simulink model "VirtualADAPT"  (Simulink 8.9)
settings: Solver=ode45  StartTime=0.0  StopTime=inf  RelTol=1e-3  EnableMultiTasking=on
PostLoadFcn:
  | FaultInjectionGUIHandle = FaultInjectionGUI;
InitFcn:
  | VirtualADAPTDataStructure
undefined symbols (not defined in the file or in its folder): ln
libraries: simulink x363
(part 2 of 26)

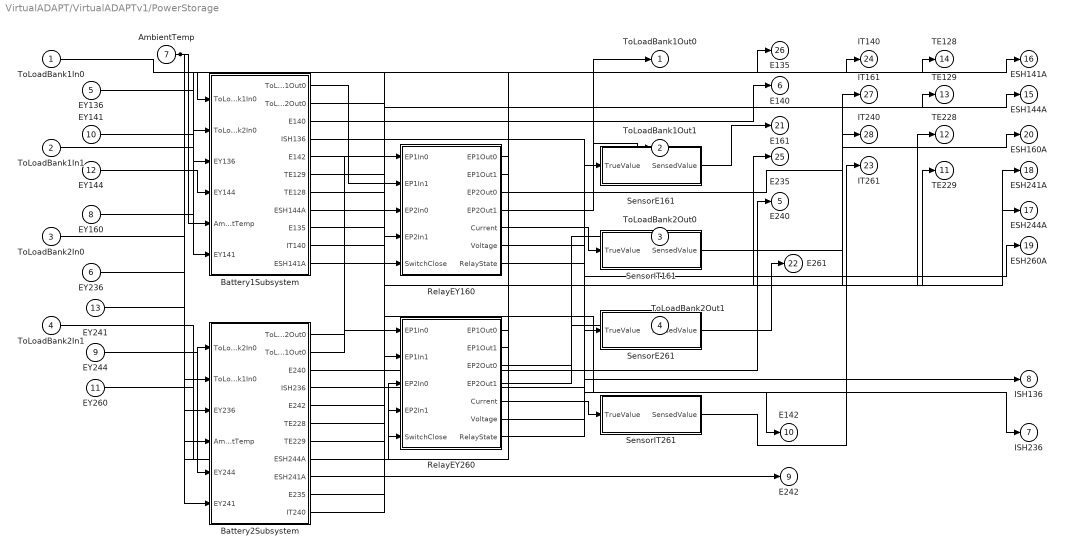
== system VirtualADAPT/VirtualADAPTv1/PowerStorage ==
  ToLoadBank1In0 : Inport
  ToLoadBank1In1 : Inport  Port=2
  ToLoadBank2In0 : Inport  Port=3
  ToLoadBank2In1 : Inport  Port=4
  EY136 : Inport  Port=5
  EY236 : Inport  Port=6
  AmbientTemp : Inport  Port=7
  EY160 : Inport  Port=8
  EY244 : Inport  Port=9
  EY141 : Inport  Port=10
  EY260 : Inport  Port=11
  EY144 : Inport  Port=12
  EY241 : Inport  Port=13
  Battery1Subsystem : Subsystem
  Battery2Subsystem : Subsystem
  RelayEY160 : Subsystem
  RelayEY260 : Subsystem
  SensorE161 : Subsystem
  SensorE261 : Subsystem
  SensorIT161 : Subsystem
  SensorIT261 : Subsystem
  ToLoadBank1Out0 : Outport
  ToLoadBank1Out1 : Outport  Port=2
  ToLoadBank2Out0 : Outport  Port=3
  ToLoadBank2Out1 : Outport  Port=4
  E240 : Outport  Port=5
  E140 : Outport  Port=6
  ISH236 : Outport  Port=7
  ISH136 : Outport  Port=8
  E242 : Outport  Port=9
  E142 : Outport  Port=10
  TE229 : Outport  Port=11
  TE228 : Outport  Port=12
  TE129 : Outport  Port=13
  TE128 : Outport  Port=14
  ESH144A : Outport  Port=15
  ESH141A : Outport  Port=16
  ESH244A : Outport  Port=17
  ESH241A : Outport  Port=18
  ESH260A : Outport  Port=19
  ESH160A : Outport  Port=20
  E161 : Outport  Port=21
  E261 : Outport  Port=22
  IT261 : Outport  Port=23
  IT140 : Outport  Port=24
  E235 : Outport  Port=25
  E135 : Outport  Port=26
  IT161 : Outport  Port=27
  IT240 : Outport  Port=28
  wires:
  Battery1Subsystem/1(ToLoadBank1Out0) -> RelayEY160/2(EP1In1)
  RelayEY160/2(EP1Out1) -> Battery1Subsystem/1(ToLoadBank1In0)
  Battery2Subsystem/1(ToLoadBank2Out0) -> RelayEY260/1(EP1In0)
  RelayEY260/1(EP1Out0) -> Battery2Subsystem/1(ToLoadBank2In0)
  Battery1Subsystem/2(ToLoadBank2Out0) -> RelayEY260/2(EP1In1)
  RelayEY260/2(EP1Out1) -> Battery1Subsystem/2(ToLoadBank2In0)
  RelayEY160/3(EP2Out0) -> ToLoadBank1Out0/1
  ToLoadBank1In0/1 -> RelayEY160/3(EP2In0)
  RelayEY160/4(EP2Out1) -> ToLoadBank1Out1/1
  ToLoadBank1In1/1 -> RelayEY160/4(EP2In1)
  Battery2Subsystem/2(ToLoadBank1Out0) -> RelayEY160/1(EP1In0)
  RelayEY160/1(EP1Out0) -> Battery2Subsystem/2(ToLoadBank1In0)
  RelayEY260/3(EP2Out0) -> ToLoadBank2Out0/1
  ToLoadBank2In0/1 -> RelayEY260/3(EP2In0)
  RelayEY260/4(EP2Out1) -> ToLoadBank2Out1/1
  ToLoadBank2In1/1 -> RelayEY260/4(EP2In1)
  Battery1Subsystem/5(E142) -> E142/1
  Battery2Subsystem/3(E240) -> E240/1
  SensorE161/1(SensedValue) -> E161/1
  Battery1Subsystem/8(ESH144A) -> ESH144A/1
  Battery1Subsystem/11(ESH141A) -> ESH141A/1
  EY260/1 -> RelayEY260/5(SwitchClose)
  SensorIT261/1(SensedValue) -> IT261/1
  EY236/1 -> Battery2Subsystem/3(EY236)
  EY136/1 -> Battery1Subsystem/3(EY136)
  Battery2Subsystem/5(E242) -> E242/1
  EY144/1 -> Battery1Subsystem/4(EY144)
  RelayEY160/5(Current) -> SensorIT161/1(TrueValue)
  RelayEY160/6(Voltage) -> SensorE161/1(TrueValue)
  RelayEY160/7(RelayState) -> ESH160A/1
  RelayEY260/5(Current) -> SensorIT261/1(TrueValue)
  RelayEY260/6(Voltage) -> SensorE261/1(TrueValue)
  RelayEY260/7(RelayState) -> ESH260A/1
  Battery2Subsystem/10(E235) -> E235/1
  SensorIT161/1(SensedValue) -> IT161/1
  SensorE261/1(SensedValue) -> E261/1
  Battery1Subsystem/10(IT140) -> IT140/1
  EY160/1 -> RelayEY160/5(SwitchClose)
  EY141/1 -> Battery1Subsystem/6(EY141)
  Battery1Subsystem/3(E140) -> E140/1
  Battery2Subsystem/11(IT240) -> IT240/1
  EY241/1 -> Battery2Subsystem/6(EY241)
  Battery1Subsystem/4(ISH136) -> ISH136/1
  Battery2Subsystem/4(ISH236) -> ISH236/1
  EY244/1 -> Battery2Subsystem/5(EY244)
  AmbientTemp/1 -> Battery2Subsystem/4(AmbientTemp), Battery1Subsystem/5(AmbientTemp)
  Battery1Subsystem/9(E135) -> E135/1
  Battery2Subsystem/8(ESH244A) -> ESH244A/1
  Battery2Subsystem/9(ESH241A) -> ESH241A/1
  Battery2Subsystem/7(TE229) -> TE229/1
  Battery2Subsystem/6(TE228) -> TE228/1
  Battery1Subsystem/6(TE129) -> TE129/1
  Battery1Subsystem/7(TE128) -> TE128/1

== system VirtualADAPT/VirtualADAPTv1/SensorTE133 ==
  TrueValue : Inport
  MSe : Subsystem  mask{FP=Nominal, FT=0, FM=0}
      mask prompts: FP "Fault Profile:"; FT "Fault Injection Time:"; FM "Fault Magnitude/Slope/Bias/StuckAtValue"
      mask TypeOptions FP: Nominal | Abrupt | Incipient | Bias | StuckAt
      mask Display: image(imread('MSe.png','BackgroundColor',[1 1 1]))
  R : Subsystem  mask{R=1, FP=Nominal, FT=0, FM=0}
      mask prompts: R "Value of R:"; FP "Fault Profile:"; FT "Fault Injection Time:"; FM "Fault Magnitude/Slope"
      mask TypeOptions FP: Nominal | Abrupt | Incipient
      mask Display: image(imread('R.png','BackgroundColor',[1 1 1]))
  TempBias : Subsystem  mask{Constant=0, FP=Nominal, FT=0, FM=0}
      mask prompts: Constant "Value of Constant:"; FP "Fault Profile:"; FT "Fault Injection Time:"; FM "Fault Magnitude/Slope"
      mask TypeOptions FP: Nominal | Abrupt | Incipient
      mask Display: image(imread('Constant.png','BackgroundColor',[1 1 1]))
  TrueValueMod : Subsystem
      mask Display: image(imread('fx.png','BackgroundColor',[1 1 1]))
  Value : Subsystem
      mask Display: image(imread('0.png','BackgroundColor',[1 1 1]))
  SensedValue : Outport
  wires:
  Value/1(y(1)) -> R/1(In)
  R/1(Out) -> Value/1(u(1))
  MSe/1(E) -> Value/2(u(2))
  Value/2(y(2)) -> MSe/1(F)
  TrueValueMod/1(y(1)) -> MSe/2(Se)
  TrueValue/1 -> TrueValueMod/1(u(1))
  TempBias/1(C) -> TrueValueMod/2(u(2))
  Value/3(O) -> SensedValue/1

== system VirtualADAPT/VirtualADAPTv1/AmbientTemp/AmbientTemp ==
  Constant : Constant  Value=Constant
  Fault Inject : Subsystem
  Fault Profile : Constant  Value=FP
  Ramp : simulink/Sources/Ramp  slope=FM  start=FT  X0=Constant
  Step : Step  Time=FT  Before=Constant  After=(1 + FM)*Constant  SampleTime=0
  C : Outport
  wires:
  Fault Inject/1(Out1) -> C/1
  Constant/1 -> Fault Inject/1(Nominal)
  Fault Profile/1 -> Fault Inject/2(Fault Profile)
  Step/1 -> Fault Inject/3(Step)
  Ramp/1 -> Fault Inject/4(Ramp)

== system VirtualADAPT/VirtualADAPTv1/EY136_OPFaultInject/Fault Inject ==
  Nominal : Inport
  Fault Profile : Inport  Port=2
  Step : Inport  Port=3
  Ramp : Inport  Port=4
  Bias : Inport  Port=5
  stuckAt : Inport  Port=6
  Switch1 : MultiPortSwitch  Inputs=5  SaturateOnIntegerOverflow=on  InputSameDT=on
  Out1 : Outport
  wires:
  Nominal/1 -> Switch1/2
  Fault Profile/1 -> Switch1/1
  Step/1 -> Switch1/3
  Ramp/1 -> Switch1/4
  Bias/1 -> Switch1/5
  stuckAt/1 -> Switch1/6
  Switch1/1 -> Out1/1

== system VirtualADAPT/VirtualADAPTv1/EY141_CLFaultInject/Fault Inject ==
  Nominal : Inport
  Fault Profile : Inport  Port=2
  Step : Inport  Port=3
  Ramp : Inport  Port=4
  Bias : Inport  Port=5
  stuckAt : Inport  Port=6
  Switch1 : MultiPortSwitch  Inputs=5  SaturateOnIntegerOverflow=on  InputSameDT=on
  Out1 : Outport
  wires:
  Nominal/1 -> Switch1/2
  Fault Profile/1 -> Switch1/1
  Step/1 -> Switch1/3
  Ramp/1 -> Switch1/4
  Bias/1 -> Switch1/5
  stuckAt/1 -> Switch1/6
  Switch1/1 -> Out1/1

== system VirtualADAPT/VirtualADAPTv1/EY144_CLFaultInject/Fault Inject ==
  Nominal : Inport
  Fault Profile : Inport  Port=2
  Step : Inport  Port=3
  Ramp : Inport  Port=4
  Bias : Inport  Port=5
  stuckAt : Inport  Port=6
  Switch1 : MultiPortSwitch  Inputs=5  SaturateOnIntegerOverflow=on  InputSameDT=on
  Out1 : Outport
  wires:
  Nominal/1 -> Switch1/2
  Fault Profile/1 -> Switch1/1
  Step/1 -> Switch1/3
  Ramp/1 -> Switch1/4
  Bias/1 -> Switch1/5
  stuckAt/1 -> Switch1/6
  Switch1/1 -> Out1/1

== system VirtualADAPT/VirtualADAPTv1/EY160_CLFaultInject/Fault Inject ==
  Nominal : Inport
  Fault Profile : Inport  Port=2
  Step : Inport  Port=3
  Ramp : Inport  Port=4
  Bias : Inport  Port=5
  stuckAt : Inport  Port=6
  Switch1 : MultiPortSwitch  Inputs=5  SaturateOnIntegerOverflow=on  InputSameDT=on
  Out1 : Outport
  wires:
  Nominal/1 -> Switch1/2
  Fault Profile/1 -> Switch1/1
  Step/1 -> Switch1/3
  Ramp/1 -> Switch1/4
  Bias/1 -> Switch1/5
  stuckAt/1 -> Switch1/6
  Switch1/1 -> Out1/1

== system VirtualADAPT/VirtualADAPTv1/EY170_CLFaultInject/Fault Inject ==
  Nominal : Inport
  Fault Profile : Inport  Port=2
  Step : Inport  Port=3
  Ramp : Inport  Port=4
  Bias : Inport  Port=5
  stuckAt : Inport  Port=6
  Switch1 : MultiPortSwitch  Inputs=5  SaturateOnIntegerOverflow=on  InputSameDT=on
  Out1 : Outport
  wires:
  Nominal/1 -> Switch1/2
  Fault Profile/1 -> Switch1/1
  Step/1 -> Switch1/3
  Ramp/1 -> Switch1/4
  Bias/1 -> Switch1/5
  stuckAt/1 -> Switch1/6
  Switch1/1 -> Out1/1

== system VirtualADAPT/VirtualADAPTv1/EY171_CLFaultInject/Fault Inject ==
  Nominal : Inport
  Fault Profile : Inport  Port=2
  Step : Inport  Port=3
  Ramp : Inport  Port=4
  Bias : Inport  Port=5
  stuckAt : Inport  Port=6
  Switch1 : MultiPortSwitch  Inputs=5  SaturateOnIntegerOverflow=on  InputSameDT=on
  Out1 : Outport
  wires:
  Nominal/1 -> Switch1/2
  Fault Profile/1 -> Switch1/1
  Step/1 -> Switch1/3
  Ramp/1 -> Switch1/4
  Bias/1 -> Switch1/5
  stuckAt/1 -> Switch1/6
  Switch1/1 -> Out1/1

== system VirtualADAPT/VirtualADAPTv1/EY172_CLFaultInject/Fault Inject ==
  Nominal : Inport
  Fault Profile : Inport  Port=2
  Step : Inport  Port=3
  Ramp : Inport  Port=4
  Bias : Inport  Port=5
  stuckAt : Inport  Port=6
  Switch1 : MultiPortSwitch  Inputs=5  SaturateOnIntegerOverflow=on  InputSameDT=on
  Out1 : Outport
  wires:
  Nominal/1 -> Switch1/2
  Fault Profile/1 -> Switch1/1
  Step/1 -> Switch1/3
  Ramp/1 -> Switch1/4
  Bias/1 -> Switch1/5
  stuckAt/1 -> Switch1/6
  Switch1/1 -> Out1/1

== system VirtualADAPT/VirtualADAPTv1/EY173_CLFaultInject/Fault Inject ==
  Nominal : Inport
  Fault Profile : Inport  Port=2
  Step : Inport  Port=3
  Ramp : Inport  Port=4
  Bias : Inport  Port=5
  stuckAt : Inport  Port=6
  Switch1 : MultiPortSwitch  Inputs=5  SaturateOnIntegerOverflow=on  InputSameDT=on
  Out1 : Outport
  wires:
  Nominal/1 -> Switch1/2
  Fault Profile/1 -> Switch1/1
  Step/1 -> Switch1/3
  Ramp/1 -> Switch1/4
  Bias/1 -> Switch1/5
  stuckAt/1 -> Switch1/6
  Switch1/1 -> Out1/1

== system VirtualADAPT/VirtualADAPTv1/EY174_CLFaultInject/Fault Inject ==
  Nominal : Inport
  Fault Profile : Inport  Port=2
  Step : Inport  Port=3
  Ramp : Inport  Port=4
  Bias : Inport  Port=5
  stuckAt : Inport  Port=6
  Switch1 : MultiPortSwitch  Inputs=5  SaturateOnIntegerOverflow=on  InputSameDT=on
  Out1 : Outport
  wires:
  Nominal/1 -> Switch1/2
  Fault Profile/1 -> Switch1/1
  Step/1 -> Switch1/3
  Ramp/1 -> Switch1/4
  Bias/1 -> Switch1/5
  stuckAt/1 -> Switch1/6
  Switch1/1 -> Out1/1

== system VirtualADAPT/VirtualADAPTv1/EY175_CLFaultInject/Fault Inject ==
  Nominal : Inport
  Fault Profile : Inport  Port=2
  Step : Inport  Port=3
  Ramp : Inport  Port=4
  Bias : Inport  Port=5
  stuckAt : Inport  Port=6
  Switch1 : MultiPortSwitch  Inputs=5  SaturateOnIntegerOverflow=on  InputSameDT=on
  Out1 : Outport
  wires:
  Nominal/1 -> Switch1/2
  Fault Profile/1 -> Switch1/1
  Step/1 -> Switch1/3
  Ramp/1 -> Switch1/4
  Bias/1 -> Switch1/5
  stuckAt/1 -> Switch1/6
  Switch1/1 -> Out1/1

== system VirtualADAPT/VirtualADAPTv1/EY183_CLFaultInject/Fault Inject ==
  Nominal : Inport
  Fault Profile : Inport  Port=2
  Step : Inport  Port=3
  Ramp : Inport  Port=4
  Bias : Inport  Port=5
  stuckAt : Inport  Port=6
  Switch1 : MultiPortSwitch  Inputs=5  SaturateOnIntegerOverflow=on  InputSameDT=on
  Out1 : Outport
  wires:
  Nominal/1 -> Switch1/2
  Fault Profile/1 -> Switch1/1
  Step/1 -> Switch1/3
  Ramp/1 -> Switch1/4
  Bias/1 -> Switch1/5
  stuckAt/1 -> Switch1/6
  Switch1/1 -> Out1/1

== system VirtualADAPT/VirtualADAPTv1/EY184_CLFaultInject/Fault Inject ==
  Nominal : Inport
  Fault Profile : Inport  Port=2
  Step : Inport  Port=3
  Ramp : Inport  Port=4
  Bias : Inport  Port=5
  stuckAt : Inport  Port=6
  Switch1 : MultiPortSwitch  Inputs=5  SaturateOnIntegerOverflow=on  InputSameDT=on
  Out1 : Outport
  wires:
  Nominal/1 -> Switch1/2
  Fault Profile/1 -> Switch1/1
  Step/1 -> Switch1/3
  Ramp/1 -> Switch1/4
  Bias/1 -> Switch1/5
  stuckAt/1 -> Switch1/6
  Switch1/1 -> Out1/1

== system VirtualADAPT/VirtualADAPTv1/EY236_OPFaultInject/Fault Inject ==
  Nominal : Inport
  Fault Profile : Inport  Port=2
  Step : Inport  Port=3
  Ramp : Inport  Port=4
  Bias : Inport  Port=5
  stuckAt : Inport  Port=6
  Switch1 : MultiPortSwitch  Inputs=5  SaturateOnIntegerOverflow=on  InputSameDT=on
  Out1 : Outport
  wires:
  Nominal/1 -> Switch1/2
  Fault Profile/1 -> Switch1/1
  Step/1 -> Switch1/3
  Ramp/1 -> Switch1/4
  Bias/1 -> Switch1/5
  stuckAt/1 -> Switch1/6
  Switch1/1 -> Out1/1

== system VirtualADAPT/VirtualADAPTv1/EY241_CLFaultInject/Fault Inject ==
  Nominal : Inport
  Fault Profile : Inport  Port=2
  Step : Inport  Port=3
  Ramp : Inport  Port=4
  Bias : Inport  Port=5
  stuckAt : Inport  Port=6
  Switch1 : MultiPortSwitch  Inputs=5  SaturateOnIntegerOverflow=on  InputSameDT=on
  Out1 : Outport
  wires:
  Nominal/1 -> Switch1/2
  Fault Profile/1 -> Switch1/1
  Step/1 -> Switch1/3
  Ramp/1 -> Switch1/4
  Bias/1 -> Switch1/5
  stuckAt/1 -> Switch1/6
  Switch1/1 -> Out1/1

== system VirtualADAPT/VirtualADAPTv1/EY244_CLFaultInject/Fault Inject ==
  Nominal : Inport
  Fault Profile : Inport  Port=2
  Step : Inport  Port=3
  Ramp : Inport  Port=4
  Bias : Inport  Port=5
  stuckAt : Inport  Port=6
  Switch1 : MultiPortSwitch  Inputs=5  SaturateOnIntegerOverflow=on  InputSameDT=on
  Out1 : Outport
  wires:
  Nominal/1 -> Switch1/2
  Fault Profile/1 -> Switch1/1
  Step/1 -> Switch1/3
  Ramp/1 -> Switch1/4
  Bias/1 -> Switch1/5
  stuckAt/1 -> Switch1/6
  Switch1/1 -> Out1/1

== system VirtualADAPT/VirtualADAPTv1/EY260_CLFaultInject/Fault Inject ==
  Nominal : Inport
  Fault Profile : Inport  Port=2
  Step : Inport  Port=3
  Ramp : Inport  Port=4
  Bias : Inport  Port=5
  stuckAt : Inport  Port=6
  Switch1 : MultiPortSwitch  Inputs=5  SaturateOnIntegerOverflow=on  InputSameDT=on
  Out1 : Outport
  wires:
  Nominal/1 -> Switch1/2
  Fault Profile/1 -> Switch1/1
  Step/1 -> Switch1/3
  Ramp/1 -> Switch1/4
  Bias/1 -> Switch1/5
  stuckAt/1 -> Switch1/6
  Switch1/1 -> Out1/1

== system VirtualADAPT/VirtualADAPTv1/EY270_CLFaultInject/Fault Inject ==
  Nominal : Inport
  Fault Profile : Inport  Port=2
  Step : Inport  Port=3
  Ramp : Inport  Port=4
  Bias : Inport  Port=5
  stuckAt : Inport  Port=6
  Switch1 : MultiPortSwitch  Inputs=5  SaturateOnIntegerOverflow=on  InputSameDT=on
  Out1 : Outport
  wires:
  Nominal/1 -> Switch1/2
  Fault Profile/1 -> Switch1/1
  Step/1 -> Switch1/3
  Ramp/1 -> Switch1/4
  Bias/1 -> Switch1/5
  stuckAt/1 -> Switch1/6
  Switch1/1 -> Out1/1

== system VirtualADAPT/VirtualADAPTv1/EY271_CLFaultInject/Fault Inject ==
  Nominal : Inport
  Fault Profile : Inport  Port=2
  Step : Inport  Port=3
  Ramp : Inport  Port=4
  Bias : Inport  Port=5
  stuckAt : Inport  Port=6
  Switch1 : MultiPortSwitch  Inputs=5  SaturateOnIntegerOverflow=on  InputSameDT=on
  Out1 : Outport
  wires:
  Nominal/1 -> Switch1/2
  Fault Profile/1 -> Switch1/1
  Step/1 -> Switch1/3
  Ramp/1 -> Switch1/4
  Bias/1 -> Switch1/5
  stuckAt/1 -> Switch1/6
  Switch1/1 -> Out1/1

== system VirtualADAPT/VirtualADAPTv1/EY272_CLFaultInject/Fault Inject ==
  Nominal : Inport
  Fault Profile : Inport  Port=2
  Step : Inport  Port=3
  Ramp : Inport  Port=4
  Bias : Inport  Port=5
  stuckAt : Inport  Port=6
  Switch1 : MultiPortSwitch  Inputs=5  SaturateOnIntegerOverflow=on  InputSameDT=on
  Out1 : Outport
  wires:
  Nominal/1 -> Switch1/2
  Fault Profile/1 -> Switch1/1
  Step/1 -> Switch1/3
  Ramp/1 -> Switch1/4
  Bias/1 -> Switch1/5
  stuckAt/1 -> Switch1/6
  Switch1/1 -> Out1/1

== system VirtualADAPT/VirtualADAPTv1/EY273_CLFaultInject/Fault Inject ==
  Nominal : Inport
  Fault Profile : Inport  Port=2
  Step : Inport  Port=3
  Ramp : Inport  Port=4
  Bias : Inport  Port=5
  stuckAt : Inport  Port=6
  Switch1 : MultiPortSwitch  Inputs=5  SaturateOnIntegerOverflow=on  InputSameDT=on
  Out1 : Outport
  wires:
  Nominal/1 -> Switch1/2
  Fault Profile/1 -> Switch1/1
  Step/1 -> Switch1/3
  Ramp/1 -> Switch1/4
  Bias/1 -> Switch1/5
  stuckAt/1 -> Switch1/6
  Switch1/1 -> Out1/1

== system VirtualADAPT/VirtualADAPTv1/EY274_CLFaultInject/Fault Inject ==
  Nominal : Inport
  Fault Profile : Inport  Port=2
  Step : Inport  Port=3
  Ramp : Inport  Port=4
  Bias : Inport  Port=5
  stuckAt : Inport  Port=6
  Switch1 : MultiPortSwitch  Inputs=5  SaturateOnIntegerOverflow=on  InputSameDT=on
  Out1 : Outport
  wires:
  Nominal/1 -> Switch1/2
  Fault Profile/1 -> Switch1/1
  Step/1 -> Switch1/3
  Ramp/1 -> Switch1/4
  Bias/1 -> Switch1/5
  stuckAt/1 -> Switch1/6
  Switch1/1 -> Out1/1

== system VirtualADAPT/VirtualADAPTv1/EY275_CLFaultInject/Fault Inject ==
  Nominal : Inport
  Fault Profile : Inport  Port=2
  Step : Inport  Port=3
  Ramp : Inport  Port=4
  Bias : Inport  Port=5
  stuckAt : Inport  Port=6
  Switch1 : MultiPortSwitch  Inputs=5  SaturateOnIntegerOverflow=on  InputSameDT=on
  Out1 : Outport
  wires:
  Nominal/1 -> Switch1/2
  Fault Profile/1 -> Switch1/1
  Step/1 -> Switch1/3
  Ramp/1 -> Switch1/4
  Bias/1 -> Switch1/5
  stuckAt/1 -> Switch1/6
  Switch1/1 -> Out1/1

== system VirtualADAPT/VirtualADAPTv1/EY283_CLFaultInject/Fault Inject ==
  Nominal : Inport
  Fault Profile : Inport  Port=2
  Step : Inport  Port=3
  Ramp : Inport  Port=4
  Bias : Inport  Port=5
  stuckAt : Inport  Port=6
  Switch1 : MultiPortSwitch  Inputs=5  SaturateOnIntegerOverflow=on  InputSameDT=on
  Out1 : Outport
  wires:
  Nominal/1 -> Switch1/2
  Fault Profile/1 -> Switch1/1
  Step/1 -> Switch1/3
  Ramp/1 -> Switch1/4
  Bias/1 -> Switch1/5
  stuckAt/1 -> Switch1/6
  Switch1/1 -> Out1/1

== system VirtualADAPT/VirtualADAPTv1/EY284_CLFaultInject/Fault Inject ==
  Nominal : Inport
  Fault Profile : Inport  Port=2
  Step : Inport  Port=3
  Ramp : Inport  Port=4
  Bias : Inport  Port=5
  stuckAt : Inport  Port=6
  Switch1 : MultiPortSwitch  Inputs=5  SaturateOnIntegerOverflow=on  InputSameDT=on
  Out1 : Outport
  wires:
  Nominal/1 -> Switch1/2
  Fault Profile/1 -> Switch1/1
  Step/1 -> Switch1/3
  Ramp/1 -> Switch1/4
  Bias/1 -> Switch1/5
  stuckAt/1 -> Switch1/6
  Switch1/1 -> Out1/1

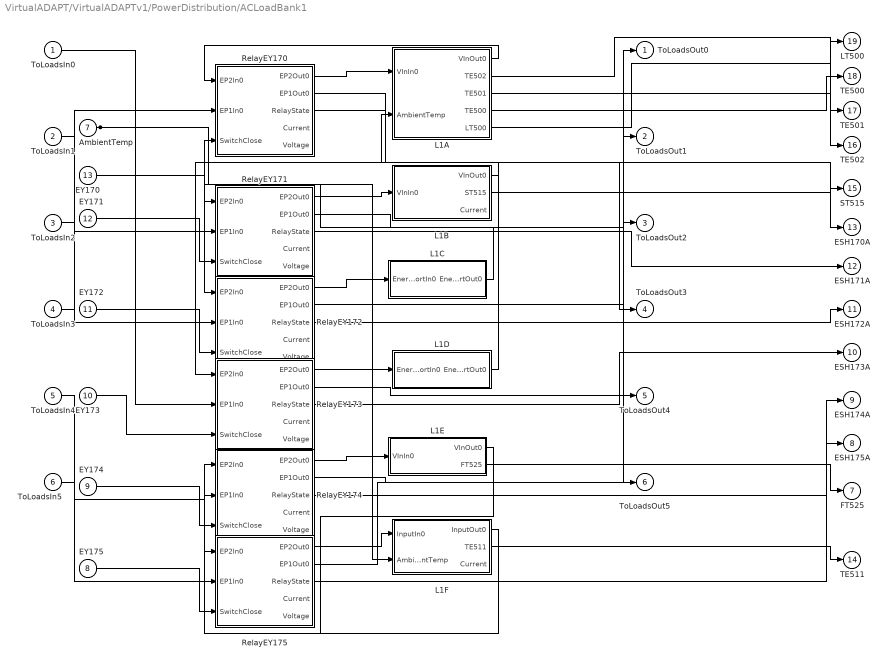
== system VirtualADAPT/VirtualADAPTv1/PowerDistribution/ACLoadBank1 ==
  ToLoadsIn0 : Inport
  ToLoadsIn1 : Inport  Port=2
  ToLoadsIn2 : Inport  Port=3
  ToLoadsIn3 : Inport  Port=4
  ToLoadsIn4 : Inport  Port=5
  ToLoadsIn5 : Inport  Port=6
  AmbientTemp : Inport  Port=7
  EY175 : Inport  Port=8
  EY174 : Inport  Port=9
  EY173 : Inport  Port=10
  EY172 : Inport  Port=11
  EY171 : Inport  Port=12
  EY170 : Inport  Port=13
  L1A : Subsystem
  L1B : Subsystem
  L1C : Subsystem
  L1D : Subsystem
  L1E : Subsystem
  L1F : Subsystem
  RelayEY170 : Subsystem
  RelayEY171 : Subsystem
  RelayEY172 : Subsystem
  RelayEY173 : Subsystem
  RelayEY174 : Subsystem
  RelayEY175 : Subsystem
  ToLoadsOut0 : Outport
  ToLoadsOut1 : Outport  Port=2
  ToLoadsOut2 : Outport  Port=3
  ToLoadsOut3 : Outport  Port=4
  ToLoadsOut4 : Outport  Port=5
  ToLoadsOut5 : Outport  Port=6
  FT525 : Outport  Port=7
  ESH175A : Outport  Port=8
  ESH174A : Outport  Port=9
  ESH173A : Outport  Port=10
  ESH172A : Outport  Port=11
  ESH171A : Outport  Port=12
  ESH170A : Outport  Port=13
  TE511 : Outport  Port=14
  ST515 : Outport  Port=15
  TE502 : Outport  Port=16
  TE501 : Outport  Port=17
  TE500 : Outport  Port=18
  LT500 : Outport  Port=19
  wires:
  RelayEY172/1(EP2Out0) -> L1C/1(EnergyPortIn0)
  L1C/1(EnergyPortOut0) -> RelayEY172/1(EP2In0)
  RelayEY173/1(EP2Out0) -> L1D/1(EnergyPortIn0)
  L1D/1(EnergyPortOut0) -> RelayEY173/1(EP2In0)
  RelayEY174/1(EP2Out0) -> L1E/1(VInIn0)
  L1E/1(VInOut0) -> RelayEY174/1(EP2In0)
  RelayEY171/1(EP2Out0) -> L1B/1(VInIn0)
  L1B/1(VInOut0) -> RelayEY171/1(EP2In0)
  ToLoadsIn0/1 -> RelayEY173/2(EP1In0)
  RelayEY173/2(EP1Out0) -> ToLoadsOut0/1
  RelayEY175/1(EP2Out0) -> L1F/1(InputIn0)
  L1F/1(InputOut0) -> RelayEY175/1(EP2In0)
  RelayEY170/1(EP2Out0) -> L1A/1(VInIn0)
  L1A/1(VInOut0) -> RelayEY170/1(EP2In0)
  ToLoadsIn1/1 -> RelayEY172/2(EP1In0)
  RelayEY172/2(EP1Out0) -> ToLoadsOut1/1
  ToLoadsIn2/1 -> RelayEY171/2(EP1In0)
  RelayEY171/2(EP1Out0) -> ToLoadsOut2/1
  ToLoadsIn3/1 -> RelayEY170/2(EP1In0)
  RelayEY170/2(EP1Out0) -> ToLoadsOut3/1
  ToLoadsIn4/1 -> RelayEY175/2(EP1In0)
  RelayEY175/2(EP1Out0) -> ToLoadsOut4/1
  ToLoadsIn5/1 -> RelayEY174/2(EP1In0)
  RelayEY174/2(EP1Out0) -> ToLoadsOut5/1
  L1E/2(FT525) -> FT525/1
  RelayEY175/3(RelayState) -> ESH175A/1
  RelayEY174/3(RelayState) -> ESH174A/1
  RelayEY173/3(RelayState) -> ESH173A/1
  RelayEY172/3(RelayState) -> ESH172A/1
  RelayEY171/3(RelayState) -> ESH171A/1
  RelayEY170/3(RelayState) -> ESH170A/1
  L1B/2(ST515) -> ST515/1
  AmbientTemp/1 -> L1F/2(AmbientTemp), L1A/2(AmbientTemp)
  L1F/2(TE511) -> TE511/1
  L1A/2(TE502) -> TE502/1
  L1A/3(TE501) -> TE501/1
  L1A/4(TE500) -> TE500/1
  L1A/5(LT500) -> LT500/1
  EY173/1 -> RelayEY173/3(SwitchClose)
  EY172/1 -> RelayEY172/3(SwitchClose)
  EY171/1 -> RelayEY171/3(SwitchClose)
  EY170/1 -> RelayEY170/3(SwitchClose)
  EY175/1 -> RelayEY175/3(SwitchClose)
  EY174/1 -> RelayEY174/3(SwitchClose)

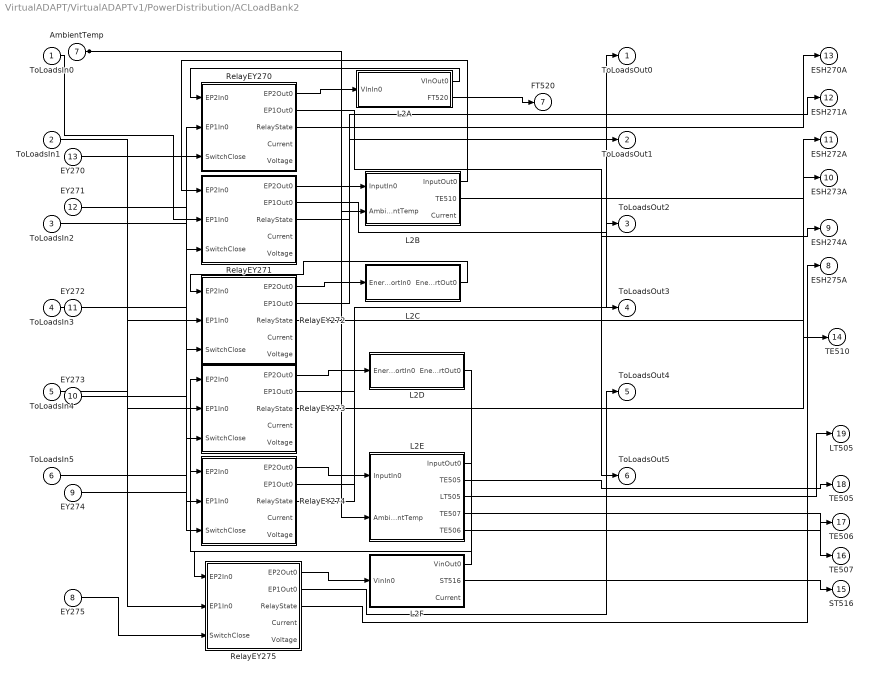
== system VirtualADAPT/VirtualADAPTv1/PowerDistribution/ACLoadBank2 ==
  ToLoadsIn0 : Inport
  ToLoadsIn1 : Inport  Port=2
  ToLoadsIn2 : Inport  Port=3
  ToLoadsIn3 : Inport  Port=4
  ToLoadsIn4 : Inport  Port=5
  ToLoadsIn5 : Inport  Port=6
  AmbientTemp : Inport  Port=7
  EY275 : Inport  Port=8
  EY274 : Inport  Port=9
  EY273 : Inport  Port=10
  EY272 : Inport  Port=11
  EY271 : Inport  Port=12
  EY270 : Inport  Port=13
  L2A : Subsystem
  L2B : Subsystem
  L2C : Subsystem
  L2D : Subsystem
  L2E : Subsystem
  L2F : Subsystem
  RelayEY270 : Subsystem
  RelayEY271 : Subsystem
  RelayEY272 : Subsystem
  RelayEY273 : Subsystem
  RelayEY274 : Subsystem
  RelayEY275 : Subsystem
  ToLoadsOut0 : Outport
  ToLoadsOut1 : Outport  Port=2
  ToLoadsOut2 : Outport  Port=3
  ToLoadsOut3 : Outport  Port=4
  ToLoadsOut4 : Outport  Port=5
  ToLoadsOut5 : Outport  Port=6
  FT520 : Outport  Port=7
  ESH275A : Outport  Port=8
  ESH274A : Outport  Port=9
  ESH273A : Outport  Port=10
  ESH272A : Outport  Port=11
  ESH271A : Outport  Port=12
  ESH270A : Outport  Port=13
  TE510 : Outport  Port=14
  ST516 : Outport  Port=15
  TE507 : Outport  Port=16
  TE506 : Outport  Port=17
  TE505 : Outport  Port=18
  LT505 : Outport  Port=19
  wires:
  RelayEY272/1(EP2Out0) -> L2C/1(EnergyPortIn0)
  L2C/1(EnergyPortOut0) -> RelayEY272/1(EP2In0)
  RelayEY273/1(EP2Out0) -> L2D/1(EnergyPortIn0)
  L2D/1(EnergyPortOut0) -> RelayEY273/1(EP2In0)
  RelayEY270/1(EP2Out0) -> L2A/1(VInIn0)
  L2A/1(VInOut0) -> RelayEY270/1(EP2In0)
  RelayEY275/1(EP2Out0) -> L2F/1(VinIn0)